simulink model "HvacMdlAirTDistbnStatFrnt"  (R2022b)
settings: Solver=FixedStepDiscrete  StartTime=0.0  StopTime=10.0  FixedStep=0.2  RelTol=1e-3
settings Model: Creator=blue.ge  LastModifiedBy=blue.ge
settings DataIOCC: SaveFormat=StructureWithTime
settings OptimizationCC: DefaultParameterBehavior=Inlined  EnhancedBackFolding=on  PassReuseOutputArgsAs=Individual arguments  ZeroExternalMemoryAtStartup=off  ZeroInternalMemoryAtStartup=off  OptimizeBlockOrder=speed  DifferentSizesBufferReuse=on  OptimizationCustomize=off  EfficientTunableParamExpr=on
settings DebuggingCC: ParameterTunabilityLossMsg=error
settings ModelReferenceCC: ModelReferenceNumInstancesAllowed=Single
settings SFSimCC: MATLABDynamicMemAlloc=off
settings RTWCC: Description=AUTOSAR  SystemTargetFile=autosar.tlc  GenCodeOnly=on  TemplateMakefile=ert_default_tmf
settings CodeAppCC: ForceParamTrailComments=on  IgnoreCustomStorageClasses=off  OperatorAnnotations=on  SimulinkDataObjDesc=on  SFDataObjDesc=on  InsertBlockDesc=on
settings STFCustomTargetCC: TargetLangStandard=C99 (ISO)  MultiwordLength=256  ParMdlRefBuildCompliant=on  CompOptLevelCompliant=on  IncludeMdlTerminateFcn=off  CombineOutputUpdateFcns=on  SuppressErrorStatus=on  ERTCustomFileBanners=on  SupportNonFinite=off  SupportComplex=off  SupportContinuousTime=off  SupportNonInlinedSFcns=off  RemoveResetFunc=on  MATLABClassNameForMDSCustomization=Simulink.SoftwareTarget.ERTCustomization  AutosarCompliant=on  UseToolchainInfoCompliant=on  LookupTableObjectStructAxisOrder=2,1,3,4,...  LUTObjectStructOrderEvenSpacing=Size,Table,Breakpoints  SystemTargetFile=autosar.tlc
settings ERTTargetCC: TargetLangStandard=C99 (ISO)  ConcurrentExecutionCompliant=off  IncludeMdlTerminateFcn=off  SuppressErrorStatus=on  SupportNonFinite=off  SupportComplex=off  ModelStepFunctionPrototypeControlCompliant=off  CPPClassGenCompliant=off  AutosarCompliant=on  LookupTableObjectStructAxisOrder=2,1,3,4,...  LUTObjectStructOrderEvenSpacing=Size,Table,Breakpoints  InlinedParameterPlacement=NonHierarchical  ERTCustomFileTemplate=example_file_process.tlc

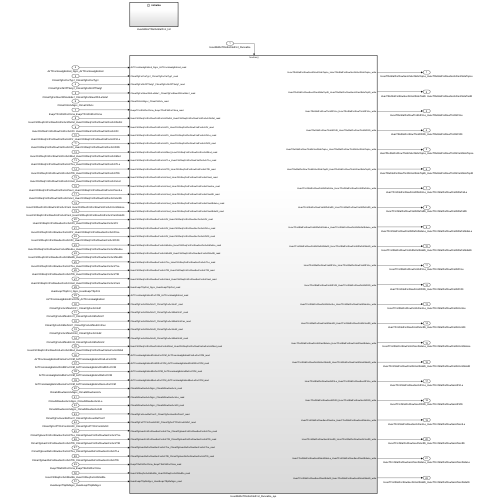
[thumbnail: Simulink's stored preview of the top-level diagram]
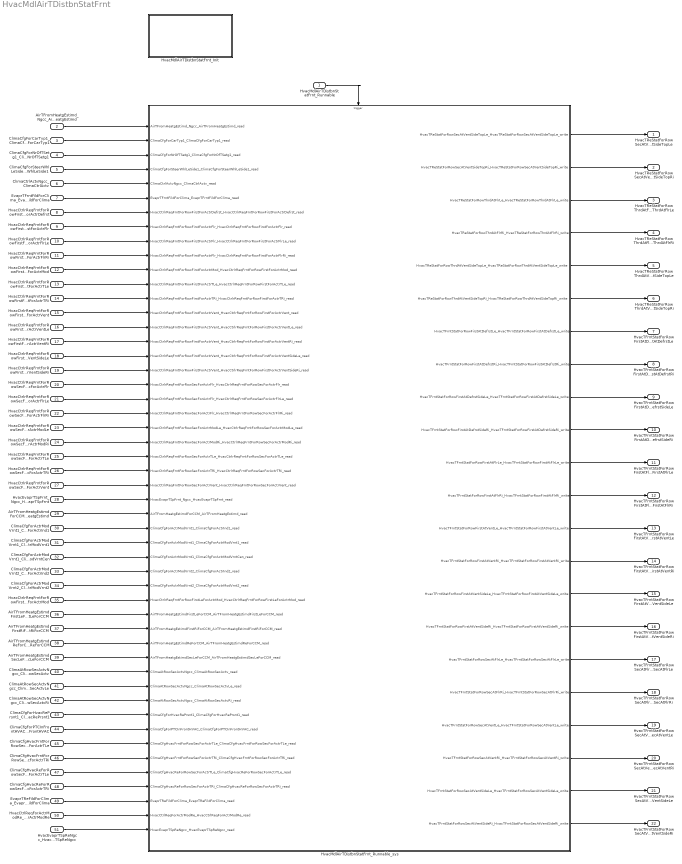
== system HvacMdlAirTDistbnStatFrnt ==
  HvacMdlAirTDistbnStatFrnt_Runnable : Inport  SampleTime=0.2  OutputFunctionCall=on
  AirTFromHeatgEstimd_Ngcc_AirTFromHeatgEstimd : Inport  Port=2  OutDataTypeStr=T30  PortDimensions=1
  ClimaCfgForCarTyp1_ClimaCfgForCarTyp1 : Inport  Port=3  OutDataTypeStr=Enum: ClimaCfgForCarTyp1  PortDimensions=1
  ClimaCfgForNrOfTSetg1_ClimaCfgForNrOfTSetg1 : Inport  Port=4  OutDataTypeStr=Enum: ClimaCfgForNrOfTSetg1  PortDimensions=1
  ClimaCfgForSteerWhlLeSide1_ClimaCfgForSteerWhlLeSide1 : Inport  Port=5  OutDataTypeStr=Boolean  PortDimensions=1
  ClimaCtrlActvNgcc_ClimaCtrlActv : Inport  Port=6  OutDataTypeStr=Boolean  PortDimensions=1
  EvaprTFrntFildForClima_EvaprTFrntFildForClima : Inport  Port=7  OutDataTypeStr=T30  PortDimensions=1
  HvacCtrlrReqFrntForRowFirstForActrDefrst_HvacCtrlrReqFrntForRowFirstForActrDefrst : Inport  Port=8  OutDataTypeStr=Perc30  PortDimensions=1
  HvacCtrlrReqFrntForRowFirstForActrFlr_HvacCtrlrReqFrntForRowFirstForActrFlr : Inport  Port=9  OutDataTypeStr=Perc30  PortDimensions=1
  HvacCtrlrReqFrntForRowFirstForActrFlr_HvacCtrlrReqFrntForRowFirstForActrFlrLe : Inport  Port=10  OutDataTypeStr=Perc30  PortDimensions=1
  HvacCtrlrReqFrntForRowFirstForActrFlr_HvacCtrlrReqFrntForRowFirstForActrFlrRi : Inport  Port=11  OutDataTypeStr=Perc30  PortDimensions=1
  HvacCtrlrReqFrntForRowFirstForActrMod_HvacCtrlrReqFrntForRowFirstForActrMod : Inport  Port=12  OutDataTypeStr=Perc30  PortDimensions=1
  HvacCtrlrReqFrntForRowFirstForActrTLe_HvacCtrlrReqFrntForRowFirstForActrTLe : Inport  Port=13  OutDataTypeStr=Perc30  PortDimensions=1
  HvacCtrlrReqFrntForRowFirstForActrTRi_HvacCtrlrReqFrntForRowFirstForActrTRi : Inport  Port=14  OutDataTypeStr=Perc30  PortDimensions=1
  HvacCtrlrReqFrntForRowFirstForActrVent_HvacCtrlrReqFrntForRowFirstForActrVent : Inport  Port=15  OutDataTypeStr=Perc30  PortDimensions=1
  HvacCtrlrReqFrntForRowFirstForActrVent_HvacCtrlrReqFrntForRowFirstForActrVentLe : Inport  Port=16  OutDataTypeStr=Perc30  PortDimensions=1
  HvacCtrlrReqFrntForRowFirstForActrVent_HvacCtrlrReqFrntForRowFirstForActrVentRi : Inport  Port=17  OutDataTypeStr=Perc30  PortDimensions=1
  HvacCtrlrReqFrntForRowFirstForActrVent_HvacCtrlrReqFrntForRowFirstForActrVentSideLe : Inport  Port=18  OutDataTypeStr=Perc30  PortDimensions=1
  HvacCtrlrReqFrntForRowFirstForActrVent_HvacCtrlrReqFrntForRowFirstForActrVentSideRi : Inport  Port=19  OutDataTypeStr=Perc30  PortDimensions=1
  HvacCtrlrReqFrntForRowSecForActrFlr_HvacCtrlrReqFrntForRowSecForActrFlr : Inport  Port=20  OutDataTypeStr=Perc30  PortDimensions=1
  HvacCtrlrReqFrntForRowSecForActrFlr_HvacCtrlrReqFrntForRowSecForActrFlrLe : Inport  Port=21  OutDataTypeStr=Perc30  PortDimensions=1
  HvacCtrlrReqFrntForRowSecForActrFlr_HvacCtrlrReqFrntForRowSecForActrFlrRi : Inport  Port=22  OutDataTypeStr=Perc30  PortDimensions=1
  HvacCtrlrReqFrntForRowSecForActrModLe_HvacCtrlrReqFrntForRowSecForActrModLe : Inport  Port=23  OutDataTypeStr=Perc30  PortDimensions=1
  HvacCtrlrReqFrntForRowSecForActrModRi_HvacCtrlrReqFrntForRowSecForActrModRi : Inport  Port=24  OutDataTypeStr=Perc30  PortDimensions=1
  HvacCtrlrReqFrntForRowSecForActrTLe_HvacCtrlrReqFrntForRowSecForActrTLe : Inport  Port=25  OutDataTypeStr=Perc30  PortDimensions=1
  HvacCtrlrReqFrntForRowSecForActrTRi_HvacCtrlrReqFrntForRowSecForActrTRi : Inport  Port=26  OutDataTypeStr=Perc30  PortDimensions=1
  HvacCtrlrReqFrntForRowSecForActrVent_HvacCtrlrReqFrntForRowSecForActrVent : Inport  Port=27  OutDataTypeStr=Perc30  PortDimensions=1
  HvacEvaprTSpFrnt_Ngcc_HvacEvaprTSpFrnt : Inport  Port=28  OutDataTypeStr=HvacEvaprTSp  PortDimensions=1
  AirTFromHeatgEstimdForCCM_AirTFromHeatgEstimd : Inport  Port=29  OutDataTypeStr=T30  PortDimensions=1
  ClimaCfgForActrModVrnt1_ClimaCfgForActrInd1 : Inport  Port=30  OutDataTypeStr=Enum: ClimaCfgForActrInd1  PortDimensions=1
  ClimaCfgForActrModVrnt1_ClimaCfgForActrModVrnt1 : Inport  Port=31  OutDataTypeStr=Enum: ClimaCfgForActrModVrnt1  PortDimensions=1
  ClimaCfgForActrModVrnt1_ClimaCfgForActrModVrntCen : Inport  Port=32  OutDataTypeStr=Enum: ClimaCfgForActrModVrntCen  PortDimensions=1
  ClimaCfgForActrModVrnt2_ClimaCfgForActrInd2 : Inport  Port=33  OutDataTypeStr=Enum: ClimaCfgForActrInd2  PortDimensions=1
  ClimaCfgForActrModVrnt2_ClimaCfgForActrModVrnt2 : Inport  Port=34  OutDataTypeStr=Enum: ClimaCfgForActrModVrnt2  PortDimensions=1
  HvacCtrlrReqFrntForRowFirstLeForActrMod_HvacCtrlrReqFrntForRowFirstLeForActrMod : Inport  Port=35  OutDataTypeStr=Perc30  PortDimensions=1
  AirTFromHeatgEstimdFirstLeForCCM_AirTFromHeatgEstimdFirstLeForCCM : Inport  Port=36  OutDataTypeStr=T30  PortDimensions=1
  AirTFromHeatgEstimdFirstRiForCCM_AirTFromHeatgEstimdFirstRiForCCM : Inport  Port=37  OutDataTypeStr=T30  PortDimensions=1
  AirTFromHeatgEstimdReForCCM_AirTFromHeatgEstimdReForCCM : Inport  Port=38  OutDataTypeStr=T30  PortDimensions=1
  AirTFromHeatgEstimdSecLeForCCM_AirTFromHeatgEstimdSecLeForCCM : Inport  Port=39  OutDataTypeStr=T30  PortDimensions=1
  ClimaAtRowSecActvNgcc_ClimaAtRowSecActv : Inport  Port=40  OutDataTypeStr=Boolean  PortDimensions=1
  ClimaAtRowSecActvNgcc_ClimaAtRowSecActvLe : Inport  Port=41  OutDataTypeStr=Boolean  PortDimensions=1
  ClimaAtRowSecActvNgcc_ClimaAtRowSecActvRi : Inport  Port=42  OutDataTypeStr=Boolean  PortDimensions=1
  ClimaCfgForHvacRePrsnt1_ClimaCfgForHvacRePrsnt1 : Inport  Port=43  OutDataTypeStr=Enum: CarCfgForHvacRe  PortDimensions=1
  ClimaCfgForPTCInFrontHVAC_ClimaCfgForPTCInFrontHVAC : Inport  Port=44  OutDataTypeStr=Enum: FrontHeaterExchanger  PortDimensions=1
  ClimaCfgHvacFrntForRowSecForActrTLe_ClimaCfgHvacFrntForRowSecForActrTLe : Inport  Port=45  OutDataTypeStr=Boolean  PortDimensions=1
  ClimaCfgHvacFrntForRowSecForActrTRi_ClimaCfgHvacFrntForRowSecForActrTRi : Inport  Port=46  OutDataTypeStr=Boolean  PortDimensions=1
  ClimaCfgHvacReForRowSecForActrTLe_ClimaCfgHvacReForRowSecForActrTLe : Inport  Port=47  OutDataTypeStr=UInt8  PortDimensions=1
  ClimaCfgHvacReForRowSecForActrTRi_ClimaCfgHvacReForRowSecForActrTRi : Inport  Port=48  OutDataTypeStr=UInt8  PortDimensions=1
  EvaprTReFildForClima_EvaprTReFildForClima : Inport  Port=49  OutDataTypeStr=T30  PortDimensions=1
  HvacCtrlReqForActrModRe_HvacCtrlReqForActrModRe : Inport  Port=50  OutDataTypeStr=Perc30  PortDimensions=1
  HvacEvaprTSpReNgcc_HvacEvaprTSpReNgcc : Inport  Port=51  OutDataTypeStr=HvacEvaprTSp  PortDimensions=1
  HvacMdlAirTDistbnStatFrnt_Init : Subsystem
  HvacMdlAirTDistbnStatFrnt_Runnable_sys : Subsystem
  HvacTReStatForRowSecAtVentSideTopLe_HvacTReStatForRowSecAtVentSideTopLe : Outport  OutDataTypeStr=T30  PortDimensions=1
  HvacTReStatForRowSecAtVentSideTopRi_HvacTReStatForRowSecAtVentSideTopRi : Outport  Port=2  OutDataTypeStr=T30  PortDimensions=1
  HvacTReStatForRowThrdAtFlrLe_HvacTReStatForRowThrdAtFlrLe : Outport  Port=3  OutDataTypeStr=T30  PortDimensions=1
  HvacTReStatForRowThrdAtFlrRi_HvacTReStatForRowThrdAtFlrRi : Outport  Port=4  OutDataTypeStr=T30  PortDimensions=1
  HvacTReStatForRowThrdAtVentSideTopLe_HvacTReStatForRowThrdAtVentSideTopLe : Outport  Port=5  OutDataTypeStr=T30  PortDimensions=1
  HvacTReStatForRowThrdAtVentSideTopRi_HvacTReStatForRowThrdAtVentSideTopRi : Outport  Port=6  OutDataTypeStr=T30  PortDimensions=1
  HvacTFrntStatForRowFirstAtDefrstLe_HvacTFrntStatForRowFirstAtDefrstLe : Outport  Port=7  OutDataTypeStr=T30  PortDimensions=1
  HvacTFrntStatForRowFirstAtDefrstRi_HvacTFrntStatForRowFirstAtDefrstRi : Outport  Port=8  OutDataTypeStr=T30  PortDimensions=1
  HvacTFrntStatForRowFirstAtDefrstSideLe_HvacTFrntStatForRowFirstAtDefrstSideLe : Outport  Port=9  OutDataTypeStr=T30  PortDimensions=1
  HvacTFrntStatForRowFirstAtDefrstSideRi_HvacTFrntStatForRowFirstAtDefrstSideRi : Outport  Port=10  OutDataTypeStr=T30  PortDimensions=1
  HvacTFrntStatForRowFirstAtFlrLe_HvacTFrntStatForRowFirstAtFlrLe : Outport  Port=11  OutDataTypeStr=T30  PortDimensions=1
  HvacTFrntStatForRowFirstAtFlrRi_HvacTFrntStatForRowFirstAtFlrRi : Outport  Port=12  OutDataTypeStr=T30  PortDimensions=1
  HvacTFrntStatForRowFirstAtVentLe_HvacTFrntStatForRowFirstAtVentLe : Outport  Port=13  OutDataTypeStr=T30  PortDimensions=1
  HvacTFrntStatForRowFirstAtVentRi_HvacTFrntStatForRowFirstAtVentRi : Outport  Port=14  OutDataTypeStr=T30  PortDimensions=1
  HvacTFrntStatForRowFirstAtVentSideLe_HvacTFrntStatForRowFirstAtVentSideLe : Outport  Port=15  OutDataTypeStr=T30  PortDimensions=1
  HvacTFrntStatForRowFirstAtVentSideRi_HvacTFrntStatForRowFirstAtVentSideRi : Outport  Port=16  OutDataTypeStr=T30  PortDimensions=1
  HvacTFrntStatForRowSecAtFlrLe_HvacTFrntStatForRowSecAtFlrLe : Outport  Port=17  OutDataTypeStr=T30  PortDimensions=1
  HvacTFrntStatForRowSecAtFlrRi_HvacTFrntStatForRowSecAtFlrRi : Outport  Port=18  OutDataTypeStr=T30  PortDimensions=1
  HvacTFrntStatForRowSecAtVentLe_HvacTFrntStatForRowSecAtVentLe : Outport  Port=19  OutDataTypeStr=T30  PortDimensions=1
  HvacTFrntStatForRowSecAtVentRi_HvacTFrntStatForRowSecAtVentRi : Outport  Port=20  OutDataTypeStr=T30  PortDimensions=1
  HvacTFrntStatForRowSecAtVentSideLe_HvacTFrntStatForRowSecAtVentSideLe : Outport  Port=21  OutDataTypeStr=T30  PortDimensions=1
  HvacTFrntStatForRowSecAtVentSideRi_HvacTFrntStatForRowSecAtVentSideRi : Outport  Port=22  OutDataTypeStr=T30  PortDimensions=1
  wires:
  AirTFromHeatgEstimd_Ngcc_AirTFromHeatgEstimd/1 -> HvacMdlAirTDistbnStatFrnt_Runnable_sys/1(AirTFromHeatgEstimd_Ngcc_AirTFromHeatgEstimd_read)
  ClimaCfgForCarTyp1_ClimaCfgForCarTyp1/1 -> HvacMdlAirTDistbnStatFrnt_Runnable_sys/2(ClimaCfgForCarTyp1_ClimaCfgForCarTyp1_read)
  ClimaCfgForNrOfTSetg1_ClimaCfgForNrOfTSetg1/1 -> HvacMdlAirTDistbnStatFrnt_Runnable_sys/3(ClimaCfgForNrOfTSetg1_ClimaCfgForNrOfTSetg1_read)
  ClimaCfgForSteerWhlLeSide1_ClimaCfgForSteerWhlLeSide1/1 -> HvacMdlAirTDistbnStatFrnt_Runnable_sys/4(ClimaCfgForSteerWhlLeSide1_ClimaCfgForSteerWhlLeSide1_read)
  ClimaCtrlActvNgcc_ClimaCtrlActv/1 -> HvacMdlAirTDistbnStatFrnt_Runnable_sys/5(ClimaCtrlActvNgcc_ClimaCtrlActv_read)
  EvaprTFrntFildForClima_EvaprTFrntFildForClima/1 -> HvacMdlAirTDistbnStatFrnt_Runnable_sys/6(EvaprTFrntFildForClima_EvaprTFrntFildForClima_read)
  HvacCtrlrReqFrntForRowFirstForActrDefrst_HvacCtrlrReqFrntForRowFirstForActrDefrst/1 -> HvacMdlAirTDistbnStatFrnt_Runnable_sys/7(HvacCtrlrReqFrntForRowFirstForActrDefrst_HvacCtrlrReqFrntForRowFirstForActrDefrst_read)
  HvacCtrlrReqFrntForRowFirstForActrFlr_HvacCtrlrReqFrntForRowFirstForActrFlr/1 -> HvacMdlAirTDistbnStatFrnt_Runnable_sys/8(HvacCtrlrReqFrntForRowFirstForActrFlr_HvacCtrlrReqFrntForRowFirstForActrFlr_read)
  HvacCtrlrReqFrntForRowFirstForActrFlr_HvacCtrlrReqFrntForRowFirstForActrFlrLe/1 -> HvacMdlAirTDistbnStatFrnt_Runnable_sys/9(HvacCtrlrReqFrntForRowFirstForActrFlr_HvacCtrlrReqFrntForRowFirstForActrFlrLe_read)
  HvacCtrlrReqFrntForRowFirstForActrFlr_HvacCtrlrReqFrntForRowFirstForActrFlrRi/1 -> HvacMdlAirTDistbnStatFrnt_Runnable_sys/10(HvacCtrlrReqFrntForRowFirstForActrFlr_HvacCtrlrReqFrntForRowFirstForActrFlrRi_read)
  HvacCtrlrReqFrntForRowFirstForActrMod_HvacCtrlrReqFrntForRowFirstForActrMod/1 -> HvacMdlAirTDistbnStatFrnt_Runnable_sys/11(HvacCtrlrReqFrntForRowFirstForActrMod_HvacCtrlrReqFrntForRowFirstForActrMod_read)
  HvacCtrlrReqFrntForRowFirstForActrTLe_HvacCtrlrReqFrntForRowFirstForActrTLe/1 -> HvacMdlAirTDistbnStatFrnt_Runnable_sys/12(HvacCtrlrReqFrntForRowFirstForActrTLe_HvacCtrlrReqFrntForRowFirstForActrTLe_read)
  HvacCtrlrReqFrntForRowFirstForActrTRi_HvacCtrlrReqFrntForRowFirstForActrTRi/1 -> HvacMdlAirTDistbnStatFrnt_Runnable_sys/13(HvacCtrlrReqFrntForRowFirstForActrTRi_HvacCtrlrReqFrntForRowFirstForActrTRi_read)
  HvacCtrlrReqFrntForRowFirstForActrVent_HvacCtrlrReqFrntForRowFirstForActrVent/1 -> HvacMdlAirTDistbnStatFrnt_Runnable_sys/14(HvacCtrlrReqFrntForRowFirstForActrVent_HvacCtrlrReqFrntForRowFirstForActrVent_read)
  HvacCtrlrReqFrntForRowFirstForActrVent_HvacCtrlrReqFrntForRowFirstForActrVentLe/1 -> HvacMdlAirTDistbnStatFrnt_Runnable_sys/15(HvacCtrlrReqFrntForRowFirstForActrVent_HvacCtrlrReqFrntForRowFirstForActrVentLe_read)
  HvacCtrlrReqFrntForRowFirstForActrVent_HvacCtrlrReqFrntForRowFirstForActrVentRi/1 -> HvacMdlAirTDistbnStatFrnt_Runnable_sys/16(HvacCtrlrReqFrntForRowFirstForActrVent_HvacCtrlrReqFrntForRowFirstForActrVentRi_read)
  HvacCtrlrReqFrntForRowFirstForActrVent_HvacCtrlrReqFrntForRowFirstForActrVentSideLe/1 -> HvacMdlAirTDistbnStatFrnt_Runnable_sys/17(HvacCtrlrReqFrntForRowFirstForActrVent_HvacCtrlrReqFrntForRowFirstForActrVentSideLe_read)
  HvacCtrlrReqFrntForRowFirstForActrVent_HvacCtrlrReqFrntForRowFirstForActrVentSideRi/1 -> HvacMdlAirTDistbnStatFrnt_Runnable_sys/18(HvacCtrlrReqFrntForRowFirstForActrVent_HvacCtrlrReqFrntForRowFirstForActrVentSideRi_read)
  HvacCtrlrReqFrntForRowSecForActrFlr_HvacCtrlrReqFrntForRowSecForActrFlr/1 -> HvacMdlAirTDistbnStatFrnt_Runnable_sys/19(HvacCtrlrReqFrntForRowSecForActrFlr_HvacCtrlrReqFrntForRowSecForActrFlr_read)
  HvacCtrlrReqFrntForRowSecForActrFlr_HvacCtrlrReqFrntForRowSecForActrFlrLe/1 -> HvacMdlAirTDistbnStatFrnt_Runnable_sys/20(HvacCtrlrReqFrntForRowSecForActrFlr_HvacCtrlrReqFrntForRowSecForActrFlrLe_read)
  HvacCtrlrReqFrntForRowSecForActrFlr_HvacCtrlrReqFrntForRowSecForActrFlrRi/1 -> HvacMdlAirTDistbnStatFrnt_Runnable_sys/21(HvacCtrlrReqFrntForRowSecForActrFlr_HvacCtrlrReqFrntForRowSecForActrFlrRi_read)
  HvacCtrlrReqFrntForRowSecForActrModLe_HvacCtrlrReqFrntForRowSecForActrModLe/1 -> HvacMdlAirTDistbnStatFrnt_Runnable_sys/22(HvacCtrlrReqFrntForRowSecForActrModLe_HvacCtrlrReqFrntForRowSecForActrModLe_read)
  HvacCtrlrReqFrntForRowSecForActrModRi_HvacCtrlrReqFrntForRowSecForActrModRi/1 -> HvacMdlAirTDistbnStatFrnt_Runnable_sys/23(HvacCtrlrReqFrntForRowSecForActrModRi_HvacCtrlrReqFrntForRowSecForActrModRi_read)
  HvacCtrlrReqFrntForRowSecForActrTLe_HvacCtrlrReqFrntForRowSecForActrTLe/1 -> HvacMdlAirTDistbnStatFrnt_Runnable_sys/24(HvacCtrlrReqFrntForRowSecForActrTLe_HvacCtrlrReqFrntForRowSecForActrTLe_read)
  HvacCtrlrReqFrntForRowSecForActrTRi_HvacCtrlrReqFrntForRowSecForActrTRi/1 -> HvacMdlAirTDistbnStatFrnt_Runnable_sys/25(HvacCtrlrReqFrntForRowSecForActrTRi_HvacCtrlrReqFrntForRowSecForActrTRi_read)
  HvacCtrlrReqFrntForRowSecForActrVent_HvacCtrlrReqFrntForRowSecForActrVent/1 -> HvacMdlAirTDistbnStatFrnt_Runnable_sys/26(HvacCtrlrReqFrntForRowSecForActrVent_HvacCtrlrReqFrntForRowSecForActrVent_read)
  HvacEvaprTSpFrnt_Ngcc_HvacEvaprTSpFrnt/1 -> HvacMdlAirTDistbnStatFrnt_Runnable_sys/27(HvacEvaprTSpFrnt_Ngcc_HvacEvaprTSpFrnt_read)
  AirTFromHeatgEstimdForCCM_AirTFromHeatgEstimd/1 -> HvacMdlAirTDistbnStatFrnt_Runnable_sys/28(AirTFromHeatgEstimdForCCM_AirTFromHeatgEstimd_read)
  ClimaCfgForActrModVrnt1_ClimaCfgForActrInd1/1 -> HvacMdlAirTDistbnStatFrnt_Runnable_sys/29(ClimaCfgForActrModVrnt1_ClimaCfgForActrInd1_read)
  ClimaCfgForActrModVrnt1_ClimaCfgForActrModVrnt1/1 -> HvacMdlAirTDistbnStatFrnt_Runnable_sys/30(ClimaCfgForActrModVrnt1_ClimaCfgForActrModVrnt1_read)
  ClimaCfgForActrModVrnt1_ClimaCfgForActrModVrntCen/1 -> HvacMdlAirTDistbnStatFrnt_Runnable_sys/31(ClimaCfgForActrModVrnt1_ClimaCfgForActrModVrntCen_read)
  ClimaCfgForActrModVrnt2_ClimaCfgForActrInd2/1 -> HvacMdlAirTDistbnStatFrnt_Runnable_sys/32(ClimaCfgForActrModVrnt2_ClimaCfgForActrInd2_read)
  ClimaCfgForActrModVrnt2_ClimaCfgForActrModVrnt2/1 -> HvacMdlAirTDistbnStatFrnt_Runnable_sys/33(ClimaCfgForActrModVrnt2_ClimaCfgForActrModVrnt2_read)
  HvacCtrlrReqFrntForRowFirstLeForActrMod_HvacCtrlrReqFrntForRowFirstLeForActrMod/1 -> HvacMdlAirTDistbnStatFrnt_Runnable_sys/34(HvacCtrlrReqFrntForRowFirstLeForActrMod_HvacCtrlrReqFrntForRowFirstLeForActrMod_read)
  AirTFromHeatgEstimdFirstLeForCCM_AirTFromHeatgEstimdFirstLeForCCM/1 -> HvacMdlAirTDistbnStatFrnt_Runnable_sys/35(AirTFromHeatgEstimdFirstLeForCCM_AirTFromHeatgEstimdFirstLeForCCM_read)
  AirTFromHeatgEstimdFirstRiForCCM_AirTFromHeatgEstimdFirstRiForCCM/1 -> HvacMdlAirTDistbnStatFrnt_Runnable_sys/36(AirTFromHeatgEstimdFirstRiForCCM_AirTFromHeatgEstimdFirstRiForCCM_read)
  AirTFromHeatgEstimdReForCCM_AirTFromHeatgEstimdReForCCM/1 -> HvacMdlAirTDistbnStatFrnt_Runnable_sys/37(AirTFromHeatgEstimdReForCCM_AirTFromHeatgEstimdReForCCM_read)
  AirTFromHeatgEstimdSecLeForCCM_AirTFromHeatgEstimdSecLeForCCM/1 -> HvacMdlAirTDistbnStatFrnt_Runnable_sys/38(AirTFromHeatgEstimdSecLeForCCM_AirTFromHeatgEstimdSecLeForCCM_read)
  ClimaAtRowSecActvNgcc_ClimaAtRowSecActv/1 -> HvacMdlAirTDistbnStatFrnt_Runnable_sys/39(ClimaAtRowSecActvNgcc_ClimaAtRowSecActv_read)
  ClimaAtRowSecActvNgcc_ClimaAtRowSecActvLe/1 -> HvacMdlAirTDistbnStatFrnt_Runnable_sys/40(ClimaAtRowSecActvNgcc_ClimaAtRowSecActvLe_read)
  ClimaAtRowSecActvNgcc_ClimaAtRowSecActvRi/1 -> HvacMdlAirTDistbnStatFrnt_Runnable_sys/41(ClimaAtRowSecActvNgcc_ClimaAtRowSecActvRi_read)
  ClimaCfgForHvacRePrsnt1_ClimaCfgForHvacRePrsnt1/1 -> HvacMdlAirTDistbnStatFrnt_Runnable_sys/42(ClimaCfgForHvacRePrsnt1_ClimaCfgForHvacRePrsnt1_read)
  ClimaCfgForPTCInFrontHVAC_ClimaCfgForPTCInFrontHVAC/1 -> HvacMdlAirTDistbnStatFrnt_Runnable_sys/43(ClimaCfgForPTCInFrontHVAC_ClimaCfgForPTCInFrontHVAC_read)
  ClimaCfgHvacFrntForRowSecForActrTLe_ClimaCfgHvacFrntForRowSecForActrTLe/1 -> HvacMdlAirTDistbnStatFrnt_Runnable_sys/44(ClimaCfgHvacFrntForRowSecForActrTLe_ClimaCfgHvacFrntForRowSecForActrTLe_read)
  ClimaCfgHvacFrntForRowSecForActrTRi_ClimaCfgHvacFrntForRowSecForActrTRi/1 -> HvacMdlAirTDistbnStatFrnt_Runnable_sys/45(ClimaCfgHvacFrntForRowSecForActrTRi_ClimaCfgHvacFrntForRowSecForActrTRi_read)
  ClimaCfgHvacReForRowSecForActrTLe_ClimaCfgHvacReForRowSecForActrTLe/1 -> HvacMdlAirTDistbnStatFrnt_Runnable_sys/46(ClimaCfgHvacReForRowSecForActrTLe_ClimaCfgHvacReForRowSecForActrTLe_read)
  ClimaCfgHvacReForRowSecForActrTRi_ClimaCfgHvacReForRowSecForActrTRi/1 -> HvacMdlAirTDistbnStatFrnt_Runnable_sys/47(ClimaCfgHvacReForRowSecForActrTRi_ClimaCfgHvacReForRowSecForActrTRi_read)
  EvaprTReFildForClima_EvaprTReFildForClima/1 -> HvacMdlAirTDistbnStatFrnt_Runnable_sys/48(EvaprTReFildForClima_EvaprTReFildForClima_read)
  HvacCtrlReqForActrModRe_HvacCtrlReqForActrModRe/1 -> HvacMdlAirTDistbnStatFrnt_Runnable_sys/49(HvacCtrlReqForActrModRe_HvacCtrlReqForActrModRe_read)
  HvacEvaprTSpReNgcc_HvacEvaprTSpReNgcc/1 -> HvacMdlAirTDistbnStatFrnt_Runnable_sys/50(HvacEvaprTSpReNgcc_HvacEvaprTSpReNgcc_read)
  HvacMdlAirTDistbnStatFrnt_Runnable_sys/1(HvacTReStatForRowSecAtVentSideTopLe_HvacTReStatForRowSecAtVentSideTopLe_write) -> HvacTReStatForRowSecAtVentSideTopLe_HvacTReStatForRowSecAtVentSideTopLe/1
  HvacMdlAirTDistbnStatFrnt_Runnable_sys/2(HvacTReStatForRowSecAtVentSideTopRi_HvacTReStatForRowSecAtVentSideTopRi_write) -> HvacTReStatForRowSecAtVentSideTopRi_HvacTReStatForRowSecAtVentSideTopRi/1
  HvacMdlAirTDistbnStatFrnt_Runnable_sys/3(HvacTReStatForRowThrdAtFlrLe_HvacTReStatForRowThrdAtFlrLe_write) -> HvacTReStatForRowThrdAtFlrLe_HvacTReStatForRowThrdAtFlrLe/1
  HvacMdlAirTDistbnStatFrnt_Runnable_sys/4(HvacTReStatForRowThrdAtFlrRi_HvacTReStatForRowThrdAtFlrRi_write) -> HvacTReStatForRowThrdAtFlrRi_HvacTReStatForRowThrdAtFlrRi/1
  HvacMdlAirTDistbnStatFrnt_Runnable_sys/5(HvacTReStatForRowThrdAtVentSideTopLe_HvacTReStatForRowThrdAtVentSideTopLe_write) -> HvacTReStatForRowThrdAtVentSideTopLe_HvacTReStatForRowThrdAtVentSideTopLe/1
  HvacMdlAirTDistbnStatFrnt_Runnable_sys/6(HvacTReStatForRowThrdAtVentSideTopRi_HvacTReStatForRowThrdAtVentSideTopRi_write) -> HvacTReStatForRowThrdAtVentSideTopRi_HvacTReStatForRowThrdAtVentSideTopRi/1
  HvacMdlAirTDistbnStatFrnt_Runnable_sys/7(HvacTFrntStatForRowFirstAtDefrstLe_HvacTFrntStatForRowFirstAtDefrstLe_write) -> HvacTFrntStatForRowFirstAtDefrstLe_HvacTFrntStatForRowFirstAtDefrstLe/1
  HvacMdlAirTDistbnStatFrnt_Runnable_sys/8(HvacTFrntStatForRowFirstAtDefrstRi_HvacTFrntStatForRowFirstAtDefrstRi_write) -> HvacTFrntStatForRowFirstAtDefrstRi_HvacTFrntStatForRowFirstAtDefrstRi/1
  HvacMdlAirTDistbnStatFrnt_Runnable_sys/9(HvacTFrntStatForRowFirstAtDefrstSideLe_HvacTFrntStatForRowFirstAtDefrstSideLe_write) -> HvacTFrntStatForRowFirstAtDefrstSideLe_HvacTFrntStatForRowFirstAtDefrstSideLe/1
  HvacMdlAirTDistbnStatFrnt_Runnable_sys/10(HvacTFrntStatForRowFirstAtDefrstSideRi_HvacTFrntStatForRowFirstAtDefrstSideRi_write) -> HvacTFrntStatForRowFirstAtDefrstSideRi_HvacTFrntStatForRowFirstAtDefrstSideRi/1
  HvacMdlAirTDistbnStatFrnt_Runnable_sys/11(HvacTFrntStatForRowFirstAtFlrLe_HvacTFrntStatForRowFirstAtFlrLe_write) -> HvacTFrntStatForRowFirstAtFlrLe_HvacTFrntStatForRowFirstAtFlrLe/1
  HvacMdlAirTDistbnStatFrnt_Runnable_sys/12(HvacTFrntStatForRowFirstAtFlrRi_HvacTFrntStatForRowFirstAtFlrRi_write) -> HvacTFrntStatForRowFirstAtFlrRi_HvacTFrntStatForRowFirstAtFlrRi/1
  HvacMdlAirTDistbnStatFrnt_Runnable_sys/13(HvacTFrntStatForRowFirstAtVentLe_HvacTFrntStatForRowFirstAtVentLe_write) -> HvacTFrntStatForRowFirstAtVentLe_HvacTFrntStatForRowFirstAtVentLe/1
  HvacMdlAirTDistbnStatFrnt_Runnable_sys/14(HvacTFrntStatForRowFirstAtVentRi_HvacTFrntStatForRowFirstAtVentRi_write) -> HvacTFrntStatForRowFirstAtVentRi_HvacTFrntStatForRowFirstAtVentRi/1
  HvacMdlAirTDistbnStatFrnt_Runnable_sys/15(HvacTFrntStatForRowFirstAtVentSideLe_HvacTFrntStatForRowFirstAtVentSideLe_write) -> HvacTFrntStatForRowFirstAtVentSideLe_HvacTFrntStatForRowFirstAtVentSideLe/1
  HvacMdlAirTDistbnStatFrnt_Runnable_sys/16(HvacTFrntStatForRowFirstAtVentSideRi_HvacTFrntStatForRowFirstAtVentSideRi_write) -> HvacTFrntStatForRowFirstAtVentSideRi_HvacTFrntStatForRowFirstAtVentSideRi/1
  HvacMdlAirTDistbnStatFrnt_Runnable_sys/17(HvacTFrntStatForRowSecAtFlrLe_HvacTFrntStatForRowSecAtFlrLe_write) -> HvacTFrntStatForRowSecAtFlrLe_HvacTFrntStatForRowSecAtFlrLe/1
  HvacMdlAirTDistbnStatFrnt_Runnable_sys/18(HvacTFrntStatForRowSecAtFlrRi_HvacTFrntStatForRowSecAtFlrRi_write) -> HvacTFrntStatForRowSecAtFlrRi_HvacTFrntStatForRowSecAtFlrRi/1
  HvacMdlAirTDistbnStatFrnt_Runnable_sys/19(HvacTFrntStatForRowSecAtVentLe_HvacTFrntStatForRowSecAtVentLe_write) -> HvacTFrntStatForRowSecAtVentLe_HvacTFrntStatForRowSecAtVentLe/1
  HvacMdlAirTDistbnStatFrnt_Runnable_sys/20(HvacTFrntStatForRowSecAtVentRi_HvacTFrntStatForRowSecAtVentRi_write) -> HvacTFrntStatForRowSecAtVentRi_HvacTFrntStatForRowSecAtVentRi/1
  HvacMdlAirTDistbnStatFrnt_Runnable_sys/21(HvacTFrntStatForRowSecAtVentSideLe_HvacTFrntStatForRowSecAtVentSideLe_write) -> HvacTFrntStatForRowSecAtVentSideLe_HvacTFrntStatForRowSecAtVentSideLe/1
  HvacMdlAirTDistbnStatFrnt_Runnable_sys/22(HvacTFrntStatForRowSecAtVentSideRi_HvacTFrntStatForRowSecAtVentSideRi_write) -> HvacTFrntStatForRowSecAtVentSideRi_HvacTFrntStatForRowSecAtVentSideRi/1
  HvacMdlAirTDistbnStatFrnt_Runnable/1 -> HvacMdlAirTDistbnStatFrnt_Runnable_sys/Trigger

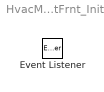
== system HvacMdlAirTDistbnStatFrnt/HvacMdlAirTDistbnStatFrnt_Init ==
  Event Listener : EventListener  EventName=reset

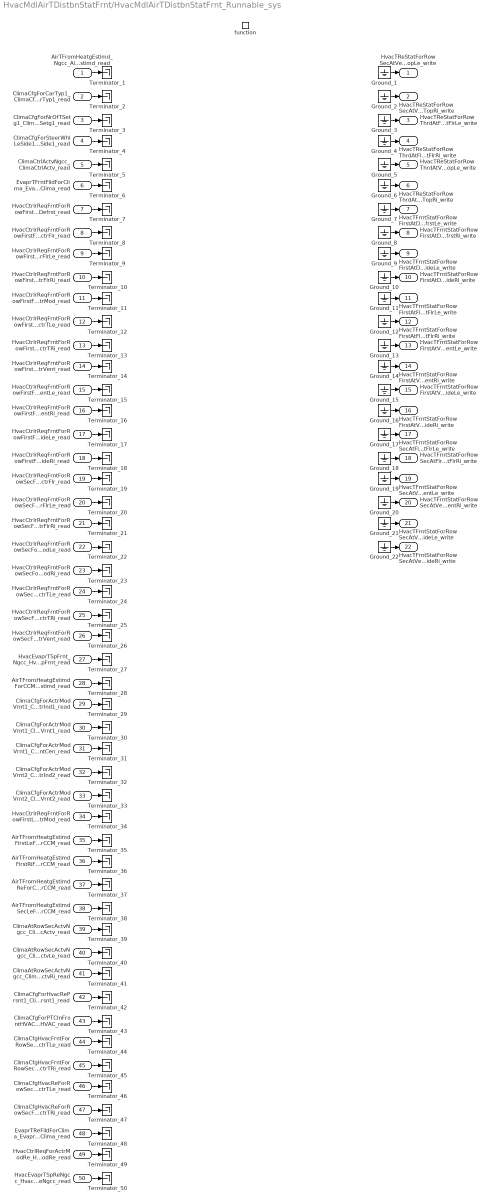
== system HvacMdlAirTDistbnStatFrnt/HvacMdlAirTDistbnStatFrnt_Runnable_sys ==
  AirTFromHeatgEstimd_Ngcc_AirTFromHeatgEstimd_read : Inport
  ClimaCfgForCarTyp1_ClimaCfgForCarTyp1_read : Inport  Port=2
  ClimaCfgForNrOfTSetg1_ClimaCfgForNrOfTSetg1_read : Inport  Port=3
  ClimaCfgForSteerWhlLeSide1_ClimaCfgForSteerWhlLeSide1_read : Inport  Port=4
  ClimaCtrlActvNgcc_ClimaCtrlActv_read : Inport  Port=5
  EvaprTFrntFildForClima_EvaprTFrntFildForClima_read : Inport  Port=6
  HvacCtrlrReqFrntForRowFirstForActrDefrst_HvacCtrlrReqFrntForRowFirstForActrDefrst_read : Inport  Port=7
  HvacCtrlrReqFrntForRowFirstForActrFlr_HvacCtrlrReqFrntForRowFirstForActrFlr_read : Inport  Port=8
  HvacCtrlrReqFrntForRowFirstForActrFlr_HvacCtrlrReqFrntForRowFirstForActrFlrLe_read : Inport  Port=9
  HvacCtrlrReqFrntForRowFirstForActrFlr_HvacCtrlrReqFrntForRowFirstForActrFlrRi_read : Inport  Port=10
  HvacCtrlrReqFrntForRowFirstForActrMod_HvacCtrlrReqFrntForRowFirstForActrMod_read : Inport  Port=11
  HvacCtrlrReqFrntForRowFirstForActrTLe_HvacCtrlrReqFrntForRowFirstForActrTLe_read : Inport  Port=12
  HvacCtrlrReqFrntForRowFirstForActrTRi_HvacCtrlrReqFrntForRowFirstForActrTRi_read : Inport  Port=13
  HvacCtrlrReqFrntForRowFirstForActrVent_HvacCtrlrReqFrntForRowFirstForActrVent_read : Inport  Port=14
  HvacCtrlrReqFrntForRowFirstForActrVent_HvacCtrlrReqFrntForRowFirstForActrVentLe_read : Inport  Port=15
  HvacCtrlrReqFrntForRowFirstForActrVent_HvacCtrlrReqFrntForRowFirstForActrVentRi_read : Inport  Port=16
  HvacCtrlrReqFrntForRowFirstForActrVent_HvacCtrlrReqFrntForRowFirstForActrVentSideLe_read : Inport  Port=17
  HvacCtrlrReqFrntForRowFirstForActrVent_HvacCtrlrReqFrntForRowFirstForActrVentSideRi_read : Inport  Port=18
  HvacCtrlrReqFrntForRowSecForActrFlr_HvacCtrlrReqFrntForRowSecForActrFlr_read : Inport  Port=19
  HvacCtrlrReqFrntForRowSecForActrFlr_HvacCtrlrReqFrntForRowSecForActrFlrLe_read : Inport  Port=20
  HvacCtrlrReqFrntForRowSecForActrFlr_HvacCtrlrReqFrntForRowSecForActrFlrRi_read : Inport  Port=21
  HvacCtrlrReqFrntForRowSecForActrModLe_HvacCtrlrReqFrntForRowSecForActrModLe_read : Inport  Port=22
  HvacCtrlrReqFrntForRowSecForActrModRi_HvacCtrlrReqFrntForRowSecForActrModRi_read : Inport  Port=23
  HvacCtrlrReqFrntForRowSecForActrTLe_HvacCtrlrReqFrntForRowSecForActrTLe_read : Inport  Port=24
  HvacCtrlrReqFrntForRowSecForActrTRi_HvacCtrlrReqFrntForRowSecForActrTRi_read : Inport  Port=25
  HvacCtrlrReqFrntForRowSecForActrVent_HvacCtrlrReqFrntForRowSecForActrVent_read : Inport  Port=26
  HvacEvaprTSpFrnt_Ngcc_HvacEvaprTSpFrnt_read : Inport  Port=27
  AirTFromHeatgEstimdForCCM_AirTFromHeatgEstimd_read : Inport  Port=28
  ClimaCfgForActrModVrnt1_ClimaCfgForActrInd1_read : Inport  Port=29
  ClimaCfgForActrModVrnt1_ClimaCfgForActrModVrnt1_read : Inport  Port=30
  ClimaCfgForActrModVrnt1_ClimaCfgForActrModVrntCen_read : Inport  Port=31
  ClimaCfgForActrModVrnt2_ClimaCfgForActrInd2_read : Inport  Port=32
  ClimaCfgForActrModVrnt2_ClimaCfgForActrModVrnt2_read : Inport  Port=33
  HvacCtrlrReqFrntForRowFirstLeForActrMod_HvacCtrlrReqFrntForRowFirstLeForActrMod_read : Inport  Port=34
  AirTFromHeatgEstimdFirstLeForCCM_AirTFromHeatgEstimdFirstLeForCCM_read : Inport  Port=35
  AirTFromHeatgEstimdFirstRiForCCM_AirTFromHeatgEstimdFirstRiForCCM_read : Inport  Port=36
  AirTFromHeatgEstimdReForCCM_AirTFromHeatgEstimdReForCCM_read : Inport  Port=37
  AirTFromHeatgEstimdSecLeForCCM_AirTFromHeatgEstimdSecLeForCCM_read : Inport  Port=38
  ClimaAtRowSecActvNgcc_ClimaAtRowSecActv_read : Inport  Port=39
  ClimaAtRowSecActvNgcc_ClimaAtRowSecActvLe_read : Inport  Port=40
  ClimaAtRowSecActvNgcc_ClimaAtRowSecActvRi_read : Inport  Port=41
  ClimaCfgForHvacRePrsnt1_ClimaCfgForHvacRePrsnt1_read : Inport  Port=42
  ClimaCfgForPTCInFrontHVAC_ClimaCfgForPTCInFrontHVAC_read : Inport  Port=43
  ClimaCfgHvacFrntForRowSecForActrTLe_ClimaCfgHvacFrntForRowSecForActrTLe_read : Inport  Port=44
  ClimaCfgHvacFrntForRowSecForActrTRi_ClimaCfgHvacFrntForRowSecForActrTRi_read : Inport  Port=45
  ClimaCfgHvacReForRowSecForActrTLe_ClimaCfgHvacReForRowSecForActrTLe_read : Inport  Port=46
  ClimaCfgHvacReForRowSecForActrTRi_ClimaCfgHvacReForRowSecForActrTRi_read : Inport  Port=47
  EvaprTReFildForClima_EvaprTReFildForClima_read : Inport  Port=48
  HvacCtrlReqForActrModRe_HvacCtrlReqForActrModRe_read : Inport  Port=49
  HvacEvaprTSpReNgcc_HvacEvaprTSpReNgcc_read : Inport  Port=50
  function : TriggerPort  TriggerType=function-call  VariantControl=(inherit)  SampleTimeType=periodic  SampleTime=-1
  Ground_{1..22} (22 alike) : Ground
  Terminator_{1..50} (50 alike) : Terminator
  HvacTReStatForRowSecAtVentSideTopLe_HvacTReStatForRowSecAtVentSideTopLe_write : Outport
      settings: EnsureOutportIsVirtual=on
  HvacTReStatForRowSecAtVentSideTopRi_HvacTReStatForRowSecAtVentSideTopRi_write : Outport  Port=2
      settings: EnsureOutportIsVirtual=on
  HvacTReStatForRowThrdAtFlrLe_HvacTReStatForRowThrdAtFlrLe_write : Outport  Port=3
      settings: EnsureOutportIsVirtual=on
  HvacTReStatForRowThrdAtFlrRi_HvacTReStatForRowThrdAtFlrRi_write : Outport  Port=4
      settings: EnsureOutportIsVirtual=on
  HvacTReStatForRowThrdAtVentSideTopLe_HvacTReStatForRowThrdAtVentSideTopLe_write : Outport  Port=5
      settings: EnsureOutportIsVirtual=on
  HvacTReStatForRowThrdAtVentSideTopRi_HvacTReStatForRowThrdAtVentSideTopRi_write : Outport  Port=6
      settings: EnsureOutportIsVirtual=on
  HvacTFrntStatForRowFirstAtDefrstLe_HvacTFrntStatForRowFirstAtDefrstLe_write : Outport  Port=7
      settings: EnsureOutportIsVirtual=on
  HvacTFrntStatForRowFirstAtDefrstRi_HvacTFrntStatForRowFirstAtDefrstRi_write : Outport  Port=8
      settings: EnsureOutportIsVirtual=on
  HvacTFrntStatForRowFirstAtDefrstSideLe_HvacTFrntStatForRowFirstAtDefrstSideLe_write : Outport  Port=9
      settings: EnsureOutportIsVirtual=on
  HvacTFrntStatForRowFirstAtDefrstSideRi_HvacTFrntStatForRowFirstAtDefrstSideRi_write : Outport  Port=10
      settings: EnsureOutportIsVirtual=on
  HvacTFrntStatForRowFirstAtFlrLe_HvacTFrntStatForRowFirstAtFlrLe_write : Outport  Port=11
      settings: EnsureOutportIsVirtual=on
  HvacTFrntStatForRowFirstAtFlrRi_HvacTFrntStatForRowFirstAtFlrRi_write : Outport  Port=12
      settings: EnsureOutportIsVirtual=on
  HvacTFrntStatForRowFirstAtVentLe_HvacTFrntStatForRowFirstAtVentLe_write : Outport  Port=13
      settings: EnsureOutportIsVirtual=on
  HvacTFrntStatForRowFirstAtVentRi_HvacTFrntStatForRowFirstAtVentRi_write : Outport  Port=14
      settings: EnsureOutportIsVirtual=on
  HvacTFrntStatForRowFirstAtVentSideLe_HvacTFrntStatForRowFirstAtVentSideLe_write : Outport  Port=15
      settings: EnsureOutportIsVirtual=on
  HvacTFrntStatForRowFirstAtVentSideRi_HvacTFrntStatForRowFirstAtVentSideRi_write : Outport  Port=16
      settings: EnsureOutportIsVirtual=on
  HvacTFrntStatForRowSecAtFlrLe_HvacTFrntStatForRowSecAtFlrLe_write : Outport  Port=17
      settings: EnsureOutportIsVirtual=on
  HvacTFrntStatForRowSecAtFlrRi_HvacTFrntStatForRowSecAtFlrRi_write : Outport  Port=18
      settings: EnsureOutportIsVirtual=on
  HvacTFrntStatForRowSecAtVentLe_HvacTFrntStatForRowSecAtVentLe_write : Outport  Port=19
      settings: EnsureOutportIsVirtual=on
  HvacTFrntStatForRowSecAtVentRi_HvacTFrntStatForRowSecAtVentRi_write : Outport  Port=20
      settings: EnsureOutportIsVirtual=on
  HvacTFrntStatForRowSecAtVentSideLe_HvacTFrntStatForRowSecAtVentSideLe_write : Outport  Port=21
      settings: EnsureOutportIsVirtual=on
  HvacTFrntStatForRowSecAtVentSideRi_HvacTFrntStatForRowSecAtVentSideRi_write : Outport  Port=22
      settings: EnsureOutportIsVirtual=on
  wires:
  AirTFromHeatgEstimd_Ngcc_AirTFromHeatgEstimd_read/1 -> Terminator_1/1
  ClimaCfgForCarTyp1_ClimaCfgForCarTyp1_read/1 -> Terminator_2/1
  ClimaCfgForNrOfTSetg1_ClimaCfgForNrOfTSetg1_read/1 -> Terminator_3/1
  ClimaCfgForSteerWhlLeSide1_ClimaCfgForSteerWhlLeSide1_read/1 -> Terminator_4/1
  ClimaCtrlActvNgcc_ClimaCtrlActv_read/1 -> Terminator_5/1
  EvaprTFrntFildForClima_EvaprTFrntFildForClima_read/1 -> Terminator_6/1
  HvacCtrlrReqFrntForRowFirstForActrDefrst_HvacCtrlrReqFrntForRowFirstForActrDefrst_read/1 -> Terminator_7/1
  HvacCtrlrReqFrntForRowFirstForActrFlr_HvacCtrlrReqFrntForRowFirstForActrFlr_read/1 -> Terminator_8/1
  HvacCtrlrReqFrntForRowFirstForActrFlr_HvacCtrlrReqFrntForRowFirstForActrFlrLe_read/1 -> Terminator_9/1
  HvacCtrlrReqFrntForRowFirstForActrFlr_HvacCtrlrReqFrntForRowFirstForActrFlrRi_read/1 -> Terminator_10/1
  HvacCtrlrReqFrntForRowFirstForActrMod_HvacCtrlrReqFrntForRowFirstForActrMod_read/1 -> Terminator_11/1
  HvacCtrlrReqFrntForRowFirstForActrTLe_HvacCtrlrReqFrntForRowFirstForActrTLe_read/1 -> Terminator_12/1
  HvacCtrlrReqFrntForRowFirstForActrTRi_HvacCtrlrReqFrntForRowFirstForActrTRi_read/1 -> Terminator_13/1
  HvacCtrlrReqFrntForRowFirstForActrVent_HvacCtrlrReqFrntForRowFirstForActrVent_read/1 -> Terminator_14/1
  HvacCtrlrReqFrntForRowFirstForActrVent_HvacCtrlrReqFrntForRowFirstForActrVentLe_read/1 -> Terminator_15/1
  HvacCtrlrReqFrntForRowFirstForActrVent_HvacCtrlrReqFrntForRowFirstForActrVentRi_read/1 -> Terminator_16/1
  HvacCtrlrReqFrntForRowFirstForActrVent_HvacCtrlrReqFrntForRowFirstForActrVentSideLe_read/1 -> Terminator_17/1
  HvacCtrlrReqFrntForRowFirstForActrVent_HvacCtrlrReqFrntForRowFirstForActrVentSideRi_read/1 -> Terminator_18/1
  HvacCtrlrReqFrntForRowSecForActrFlr_HvacCtrlrReqFrntForRowSecForActrFlr_read/1 -> Terminator_19/1
  HvacCtrlrReqFrntForRowSecForActrFlr_HvacCtrlrReqFrntForRowSecForActrFlrLe_read/1 -> Terminator_20/1
  HvacCtrlrReqFrntForRowSecForActrFlr_HvacCtrlrReqFrntForRowSecForActrFlrRi_read/1 -> Terminator_21/1
  HvacCtrlrReqFrntForRowSecForActrModLe_HvacCtrlrReqFrntForRowSecForActrModLe_read/1 -> Terminator_22/1
  HvacCtrlrReqFrntForRowSecForActrModRi_HvacCtrlrReqFrntForRowSecForActrModRi_read/1 -> Terminator_23/1
  HvacCtrlrReqFrntForRowSecForActrTLe_HvacCtrlrReqFrntForRowSecForActrTLe_read/1 -> Terminator_24/1
  HvacCtrlrReqFrntForRowSecForActrTRi_HvacCtrlrReqFrntForRowSecForActrTRi_read/1 -> Terminator_25/1
  HvacCtrlrReqFrntForRowSecForActrVent_HvacCtrlrReqFrntForRowSecForActrVent_read/1 -> Terminator_26/1
  HvacEvaprTSpFrnt_Ngcc_HvacEvaprTSpFrnt_read/1 -> Terminator_27/1
  AirTFromHeatgEstimdForCCM_AirTFromHeatgEstimd_read/1 -> Terminator_28/1
  ClimaCfgForActrModVrnt1_ClimaCfgForActrInd1_read/1 -> Terminator_29/1
  ClimaCfgForActrModVrnt1_ClimaCfgForActrModVrnt1_read/1 -> Terminator_30/1
  ClimaCfgForActrModVrnt1_ClimaCfgForActrModVrntCen_read/1 -> Terminator_31/1
  ClimaCfgForActrModVrnt2_ClimaCfgForActrInd2_read/1 -> Terminator_32/1
  ClimaCfgForActrModVrnt2_ClimaCfgForActrModVrnt2_read/1 -> Terminator_33/1
  HvacCtrlrReqFrntForRowFirstLeForActrMod_HvacCtrlrReqFrntForRowFirstLeForActrMod_read/1 -> Terminator_34/1
  AirTFromHeatgEstimdFirstLeForCCM_AirTFromHeatgEstimdFirstLeForCCM_read/1 -> Terminator_35/1
  AirTFromHeatgEstimdFirstRiForCCM_AirTFromHeatgEstimdFirstRiForCCM_read/1 -> Terminator_36/1
  AirTFromHeatgEstimdReForCCM_AirTFromHeatgEstimdReForCCM_read/1 -> Terminator_37/1
  AirTFromHeatgEstimdSecLeForCCM_AirTFromHeatgEstimdSecLeForCCM_read/1 -> Terminator_38/1
  ClimaAtRowSecActvNgcc_ClimaAtRowSecActv_read/1 -> Terminator_39/1
  ClimaAtRowSecActvNgcc_ClimaAtRowSecActvLe_read/1 -> Terminator_40/1
  ClimaAtRowSecActvNgcc_ClimaAtRowSecActvRi_read/1 -> Terminator_41/1
  ClimaCfgForHvacRePrsnt1_ClimaCfgForHvacRePrsnt1_read/1 -> Terminator_42/1
  ClimaCfgForPTCInFrontHVAC_ClimaCfgForPTCInFrontHVAC_read/1 -> Terminator_43/1
  ClimaCfgHvacFrntForRowSecForActrTLe_ClimaCfgHvacFrntForRowSecForActrTLe_read/1 -> Terminator_44/1
  ClimaCfgHvacFrntForRowSecForActrTRi_ClimaCfgHvacFrntForRowSecForActrTRi_read/1 -> Terminator_45/1
  ClimaCfgHvacReForRowSecForActrTLe_ClimaCfgHvacReForRowSecForActrTLe_read/1 -> Terminator_46/1
  ClimaCfgHvacReForRowSecForActrTRi_ClimaCfgHvacReForRowSecForActrTRi_read/1 -> Terminator_47/1
  EvaprTReFildForClima_EvaprTReFildForClima_read/1 -> Terminator_48/1
  HvacCtrlReqForActrModRe_HvacCtrlReqForActrModRe_read/1 -> Terminator_49/1
  HvacEvaprTSpReNgcc_HvacEvaprTSpReNgcc_read/1 -> Terminator_50/1
  Ground_1/1 -> HvacTReStatForRowSecAtVentSideTopLe_HvacTReStatForRowSecAtVentSideTopLe_write/1
  Ground_2/1 -> HvacTReStatForRowSecAtVentSideTopRi_HvacTReStatForRowSecAtVentSideTopRi_write/1
  Ground_3/1 -> HvacTReStatForRowThrdAtFlrLe_HvacTReStatForRowThrdAtFlrLe_write/1
  Ground_4/1 -> HvacTReStatForRowThrdAtFlrRi_HvacTReStatForRowThrdAtFlrRi_write/1
  Ground_5/1 -> HvacTReStatForRowThrdAtVentSideTopLe_HvacTReStatForRowThrdAtVentSideTopLe_write/1
  Ground_6/1 -> HvacTReStatForRowThrdAtVentSideTopRi_HvacTReStatForRowThrdAtVentSideTopRi_write/1
  Ground_7/1 -> HvacTFrntStatForRowFirstAtDefrstLe_HvacTFrntStatForRowFirstAtDefrstLe_write/1
  Ground_8/1 -> HvacTFrntStatForRowFirstAtDefrstRi_HvacTFrntStatForRowFirstAtDefrstRi_write/1
  Ground_9/1 -> HvacTFrntStatForRowFirstAtDefrstSideLe_HvacTFrntStatForRowFirstAtDefrstSideLe_write/1
  Ground_10/1 -> HvacTFrntStatForRowFirstAtDefrstSideRi_HvacTFrntStatForRowFirstAtDefrstSideRi_write/1
  Ground_11/1 -> HvacTFrntStatForRowFirstAtFlrLe_HvacTFrntStatForRowFirstAtFlrLe_write/1
  Ground_12/1 -> HvacTFrntStatForRowFirstAtFlrRi_HvacTFrntStatForRowFirstAtFlrRi_write/1
  Ground_13/1 -> HvacTFrntStatForRowFirstAtVentLe_HvacTFrntStatForRowFirstAtVentLe_write/1
  Ground_14/1 -> HvacTFrntStatForRowFirstAtVentRi_HvacTFrntStatForRowFirstAtVentRi_write/1
  Ground_15/1 -> HvacTFrntStatForRowFirstAtVentSideLe_HvacTFrntStatForRowFirstAtVentSideLe_write/1
  Ground_16/1 -> HvacTFrntStatForRowFirstAtVentSideRi_HvacTFrntStatForRowFirstAtVentSideRi_write/1
  Ground_17/1 -> HvacTFrntStatForRowSecAtFlrLe_HvacTFrntStatForRowSecAtFlrLe_write/1
  Ground_18/1 -> HvacTFrntStatForRowSecAtFlrRi_HvacTFrntStatForRowSecAtFlrRi_write/1
  Ground_19/1 -> HvacTFrntStatForRowSecAtVentLe_HvacTFrntStatForRowSecAtVentLe_write/1
  Ground_20/1 -> HvacTFrntStatForRowSecAtVentRi_HvacTFrntStatForRowSecAtVentRi_write/1
  Ground_21/1 -> HvacTFrntStatForRowSecAtVentSideLe_HvacTFrntStatForRowSecAtVentSideLe_write/1
  Ground_22/1 -> HvacTFrntStatForRowSecAtVentSideRi_HvacTFrntStatForRowSecAtVentSideRi_write/1
